simulink model "PrkgClimaEgyMgr"  (R2022b)
settings: Solver=FixedStepDiscrete  StartTime=0.0  StopTime=10.0  FixedStep=0.2  RelTol=1e-3
settings Model: Creator=blue.ge  LastModifiedBy=blue.ge
settings DataIOCC: SaveFormat=StructureWithTime
settings OptimizationCC: DefaultParameterBehavior=Inlined  EnhancedBackFolding=on  PassReuseOutputArgsAs=Individual arguments  ZeroExternalMemoryAtStartup=off  ZeroInternalMemoryAtStartup=off  OptimizeBlockOrder=speed  DifferentSizesBufferReuse=on  OptimizationCustomize=off  EfficientTunableParamExpr=on
settings DebuggingCC: ParameterTunabilityLossMsg=error
settings ModelReferenceCC: ModelReferenceNumInstancesAllowed=Single
settings SFSimCC: MATLABDynamicMemAlloc=off
settings RTWCC: Description=AUTOSAR  SystemTargetFile=autosar.tlc  GenCodeOnly=on  TemplateMakefile=ert_default_tmf
settings CodeAppCC: ForceParamTrailComments=on  IgnoreCustomStorageClasses=off  OperatorAnnotations=on  SimulinkDataObjDesc=on  SFDataObjDesc=on  InsertBlockDesc=on
settings STFCustomTargetCC: TargetLangStandard=C99 (ISO)  MultiwordLength=256  ParMdlRefBuildCompliant=on  CompOptLevelCompliant=on  IncludeMdlTerminateFcn=off  CombineOutputUpdateFcns=on  SuppressErrorStatus=on  ERTCustomFileBanners=on  SupportNonFinite=off  SupportComplex=off  SupportContinuousTime=off  SupportNonInlinedSFcns=off  RemoveResetFunc=on  MATLABClassNameForMDSCustomization=Simulink.SoftwareTarget.ERTCustomization  AutosarCompliant=on  UseToolchainInfoCompliant=on  LookupTableObjectStructAxisOrder=2,1,3,4,...  LUTObjectStructOrderEvenSpacing=Size,Table,Breakpoints  SystemTargetFile=autosar.tlc
settings ERTTargetCC: TargetLangStandard=C99 (ISO)  ConcurrentExecutionCompliant=off  IncludeMdlTerminateFcn=off  SuppressErrorStatus=on  SupportNonFinite=off  SupportComplex=off  ModelStepFunctionPrototypeControlCompliant=off  CPPClassGenCompliant=off  AutosarCompliant=on  LookupTableObjectStructAxisOrder=2,1,3,4,...  LUTObjectStructOrderEvenSpacing=Size,Table,Breakpoints  InlinedParameterPlacement=NonHierarchical  ERTCustomFileTemplate=example_file_process.tlc

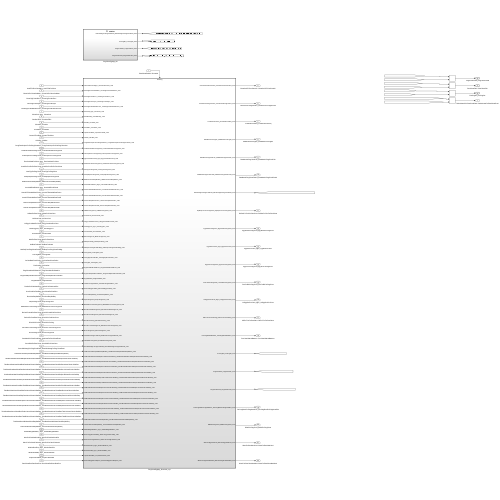
[thumbnail: Simulink's stored preview of the top-level diagram]
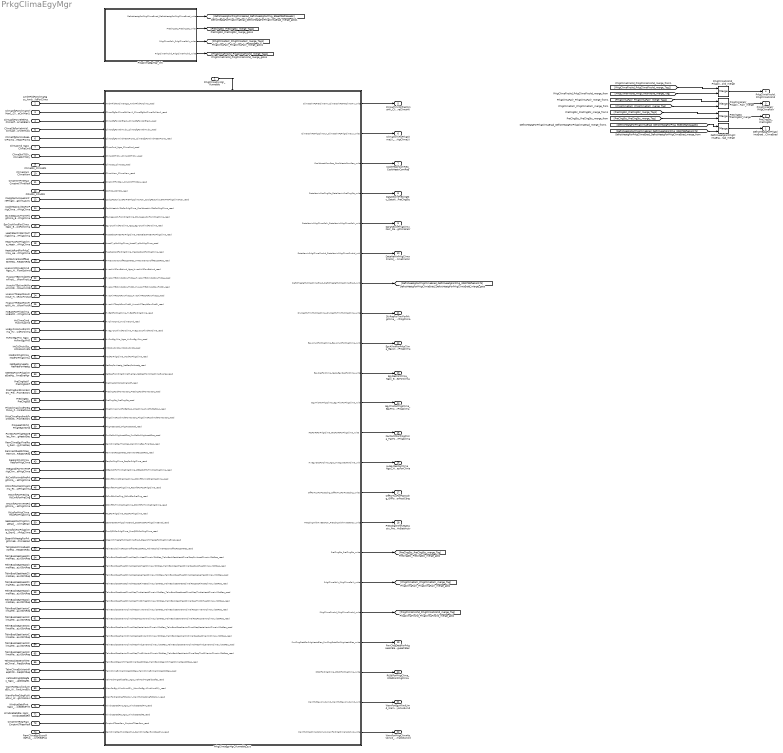
== system PrkgClimaEgyMgr ==
  PrkgClimaEgyMgr_Runnable : Inport  SampleTime=0.2  OutputFunctionCall=on
  AmbTFildForClimaNgcc_AmbTFildForClima : Inport  Port=2  OutDataTypeStr=T30  PortDimensions=1
  ClimaCfgForClimaCtrlMan1_ClimaCfgForClimaCtrlMan1 : Inport  Port=3  OutDataTypeStr=Boolean  PortDimensions=1
  ClimaCfgForVehElec1_ClimaCfgForVehElec1 : Inport  Port=4  OutDataTypeStr=Boolean  PortDimensions=1
  ClimaCfgForVehHyb1_ClimaCfgForVehHyb1 : Inport  Port=5  OutDataTypeStr=Boolean  PortDimensions=1
  ClimaCfgForWindHeatrPrsnt1_ClimaCfgForWindHeatrPrsnt1 : Inport  Port=6  OutDataTypeStr=Boolean  PortDimensions=1
  ClimaCmd_Ngcc_ClimaCmd : Inport  Port=7  OutDataTypeStr=Enum: OkNotOk  PortDimensions=1
  ClimaStrtTiDly_ClimaStrtTiDly : Inport  Port=8  OutDataTypeStr=Ti39  PortDimensions=1
  ClimaSts_ClimaSts : Inport  Port=9  OutDataTypeStr=Enum: ClimaSts  PortDimensions=1
  ClimaWarn_ClimaWarn : Inport  Port=10  OutDataTypeStr=Enum: ClimaWarn  PortDimensions=1
  CmptmtTFrntRaw_CmptmtTFrntRaw : Inport  Port=11  OutDataTypeStr=Bus: CmptmtTFrnt  PortDimensions=1  BusOutputAsStruct=on
  COHSts_COHSts : Inport  Port=12  OutDataTypeStr=Enum: CabinOHSts  PortDimensions=1
  CoolgFastUniqueForPrePrkgClimaActv_CoolgFastUniqueForPrePrkgClimaActv : Inport  Port=13  OutDataTypeStr=Boolean  PortDimensions=1
  CooltHeatrAvlStsForPrkgClima_CooltHeatrAvlStsForPrkgClima : Inport  Port=14  OutDataTypeStr=Bus: CooltHeatrAvlSts1  PortDimensions=1  BusOutputAsStruct=on
  EcuKeepActvFromPrkgClima_EcuKeepActvFromPrkgClima : Inport  Port=15  OutDataTypeStr=Boolean  PortDimensions=1
  EgyCnsAllwdForClima_Ngcc_EgyCnsAllwdForClima : Inport  Port=16  OutDataTypeStr=Egy2  PortDimensions=1
  HeatrElecFrntErrForPrkgClima_HeatrElecFrntErrForPrkgClima : Inport  Port=17  OutDataTypeStr=Boolean  PortDimensions=1
  HeatrTypForPrkgClima_HeatrTypForPrkgClima : Inport  Port=18  OutDataTypeStr=Enum: HeatrTyp  PortDimensions=1
  HeatUpRqrdForPrkgClima_HeatUpRqrdForPrkgClima : Inport  Port=19  OutDataTypeStr=Boolean  PortDimensions=1
  HMIExtVentonoffReqStrtReq_HMIExtVentonoffReqStrtReq : Inport  Port=20  OutDataTypeStr=Enum: OnOffNoReq  PortDimensions=1
  HvacAirMFlowEstimd_Ngcc_HvacAirMFlowEstimd : Inport  Port=21  OutMin=0  OutMax=1000  OutDataTypeStr=MFlow1  PortDimensions=1
  HvacAirTEstimdAtRowFirstLe_HvacAirTEstimdAtRowFirstLe : Inport  Port=22  OutDataTypeStr=T30  PortDimensions=1
  HvacAirTEstimdAtRowFirstRi_HvacAirTEstimdAtRowFirstRi : Inport  Port=23  OutDataTypeStr=T30  PortDimensions=1
  HvacAirTReqAtRowFirstLe_HvacAirTReqAtRowFirstLe : Inport  Port=24  OutDataTypeStr=T30  PortDimensions=1
  HvacAirTReqAtRowFirstRi_HvacAirTReqAtRowFirstRi : Inport  Port=25  OutDataTypeStr=T30  PortDimensions=1
  HvBattForPrkgClima_HvBattForPrkgClima : Inport  Port=26  OutDataTypeStr=Boolean  PortDimensions=1
  HvClimaCmd_HvClimaCmd : Inport  Port=27  OutDataTypeStr=Enum: HVBattChargnCmd  PortDimensions=1
  HvEgyCnsAllwdForClima_HvEgyCnsAllwdForClima : Inport  Port=28  OutDataTypeStr=HvEgyDesForClima  PortDimensions=1
  HvPwrEgyPrio_Ngcc_HvPwrEgyPrio : Inport  Port=29  OutDataTypeStr=Enum: HvPwrEgyPrio  PortDimensions=1
  IntCo2ActvSts_IntCo2ActvSts : Inport  Port=30  OutDataTypeStr=Enum: IntCo2Sts  PortDimensions=1
  ModForPrkgClima_ModForPrkgClima : Inport  Port=31  OutDataTypeStr=Enum: PrkgClimaMod  PortDimensions=1
  NetReqForHeatg_NetReqForHeatg : Inport  Port=32  OutDataTypeStr=Boolean  PortDimensions=1
  NetReqFromPrkgClimaEveMgr_NetReqFromPrkgClimaEveMgr : Inport  Port=33  OutDataTypeStr=Boolean  PortDimensions=1
  PreClngNotif_PreClngNotif : Inport  Port=34  OutDataTypeStr=Enum: PreClngNotif  PortDimensions=1
  PreClngRqrdFromExtSrc_PreClngRqrdFromExtSrc : Inport  Port=35  OutDataTypeStr=Enum: PreCdngReqFromExtSrc  PortDimensions=1
  PreClngSts_PreClngSts : Inport  Port=36  OutDataTypeStr=Enum: PreClngSts  PortDimensions=1
  PrkgClimaAllwdForBattULo_PrkgClimaAllwdForBattULo : Inport  Port=37  OutDataTypeStr=Boolean  PortDimensions=1
  PrkgClimaRqrdImdtFromExtSrc_PrkgClimaRqrdImdtFromExtSrc : Inport  Port=38  OutDataTypeStr=Enum: PreCdngReqFromExtSrc  PortDimensions=1
  PrkgHeatrStrtd_PrkgHeatrStrtd : Inport  Port=39  OutDataTypeStr=Boolean  PortDimensions=1
  PwrStsForPrkgHeatrElec_PwrStsForPrkgHeatrElec : Inport  Port=40  OutDataTypeStr=Boolean  PortDimensions=1
  RemClimaEgyFinalReq_RemClimaEgyFinalReq : Inport  Port=41  OutDataTypeStr=UInt32  PortDimensions=1
  RemVentReqStrtReq_RemVentReqStrtReq : Inport  Port=42  OutDataTypeStr=Boolean  PortDimensions=1
  ReqForPrkgClima_ReqForPrkgClima : Inport  Port=43  OutDataTypeStr=Enum: PrkgClimaReqSts  PortDimensions=1
  RtBasSdlForTmrPrePrkgClima_RtBasSdlForTmrPrePrkgClima : Inport  Port=44  OutDataTypeStr=Ti33  PortDimensions=1
  RtCmftForImdtPrePrkgClima_RtCmftForImdtPrePrkgClima : Inport  Port=45  OutDataTypeStr=Ti33  PortDimensions=1
  RtCmftForPostPrkgClima_RtCmftForPostPrkgClima : Inport  Port=46  OutDataTypeStr=Ti33  PortDimensions=1
  RtCmftForPreClng_RtCmftForPreClng : Inport  Port=47  OutDataTypeStr=Ti33  PortDimensions=1
  RtCmftForTmrPrePrkgClima_RtCmftForTmrPrePrkgClima : Inport  Port=48  OutDataTypeStr=Ti33  PortDimensions=1
  RtLeForPrkgClima_RtLeForPrkgClima : Inport  Port=49  OutDataTypeStr=Ti33  PortDimensions=1
  SeatHeatForPrkgClimaEnad_SeatHeatForPrkgClimaEnad : Inport  Port=50  OutDataTypeStr=Enum: SeatHeatDurgClimaEnad  PortDimensions=1
  SnsrQfOkForPrkgClima_SnsrQfOkForPrkgClima : Inport  Port=51  OutDataTypeStr=Boolean  PortDimensions=1
  SteerWhlHeatgForPrkgClimaEnad_SteerWhlHeatgForPrkgClimaEnad : Inport  Port=52  OutDataTypeStr=Boolean  PortDimensions=1
  TelmBookClimaReqOnOffNoReqStrtReq_TelmBookClimaReqOnOffNoReqStrtReq : Inport  Port=53  OutDataTypeStr=Enum: NoReqOnOff  PortDimensions=1
  TelmBookSeatHeatClimalReqDrvHeatClimaLvlStrtReq_TelmBookSeatHeatClimalReqDrvHeatClimaLvlStrtReq : Inport  Port=54  OutDataTypeStr=Enum: TelmSeatClimaLvl  PortDimensions=1
  TelmBookSeatHeatClimalReqPassHeatClimaLvlStrtReq_TelmBookSeatHeatClimalReqPassHeatClimaLvlStrtReq : Inport  Port=55  OutDataTypeStr=Enum: TelmSeatClimaLvl  PortDimensions=1
  TelmBookSeatHeatClimalReqSecLeHeatClimaLvlStrtReq_TelmBookSeatHeatClimalReqSecLeHeatClimaLvlStrtReq : Inport  Port=56  OutDataTypeStr=Enum: TelmSeatClimaLvl  PortDimensions=1
  TelmBookSeatHeatClimalReqSecRiHeatClimaLvlStrtReq_TelmBookSeatHeatClimalReqSecRiHeatClimaLvlStrtReq : Inport  Port=57  OutDataTypeStr=Enum: TelmSeatClimaLvl  PortDimensions=1
  TelmBookSeatHeatClimalReqThrdLeHeatClimaLvlStrtReq_TelmBookSeatHeatClimalReqThrdLeHeatClimaLvlStrtReq : Inport  Port=58  OutDataTypeStr=Enum: TelmSeatClimaLvl  PortDimensions=1
  TelmBookSeatHeatClimalReqThrdRiHeatClimaLvlStrtReq_TelmBookSeatHeatClimalReqThrdRiHeatClimaLvlStrtReq : Inport  Port=59  OutDataTypeStr=Enum: TelmSeatClimaLvl  PortDimensions=1
  TelmBookSeatVentnClimalReqDrvVentnClimaLvlStrtReq_TelmBookSeatVentnClimalReqDrvVentnClimaLvlStrtReq : Inport  Port=60  OutDataTypeStr=Enum: TelmSeatClimaLvl  PortDimensions=1
  TelmBookSeatVentnClimalReqPassVentnClimaLvlStrtReq_TelmBookSeatVentnClimalReqPassVentnClimaLvlStrtReq : Inport  Port=61  OutDataTypeStr=Enum: TelmSeatClimaLvl  PortDimensions=1
  TelmBookSeatVentnClimalReqSecLeVentnClimaLvlStrtReq_TelmBookSeatVentnClimalReqSecLeVentnClimaLvlStrtReq : Inport  Port=62  OutDataTypeStr=Enum: TelmSeatClimaLvl  PortDimensions=1
  TelmBookSeatVentnClimalReqSecRiVentnClimaLvlStrtReq_TelmBookSeatVentnClimalReqSecRiVentnClimaLvlStrtReq : Inport  Port=63  OutDataTypeStr=Enum: TelmSeatClimaLvl  PortDimensions=1
  TelmBookSeatVentnClimalReqThrdLeVentnClimaLvlStrtReq_TelmBookSeatVentnClimalReqThrdLeVentnClimaLvlStrtReq : Inport  Port=64  OutDataTypeStr=Enum: TelmSeatClimaLvl  PortDimensions=1
  TelmBookSeatVentnClimalReqThrdRiVentnClimaLvlStrtReq_TelmBookSeatVentnClimalReqThrdRiVentnClimaLvlStrtReq : Inport  Port=65  OutDataTypeStr=Enum: TelmSeatClimaLvl  PortDimensions=1
  TelmBookSteerWhlHeatClimalReqStrtReq_TelmBookSteerWhlHeatClimalReqStrtReq : Inport  Port=66  OutDataTypeStr=Enum: OnOff1  PortDimensions=1
  TelmClimaExtVentnReqStrtReq_TelmClimaExtVentnReqStrtReq : Inport  Port=67  OutDataTypeStr=Enum: OnOffNoReq  PortDimensions=1
  VehModMngtGlbSafe1_Ngcc_VehModMngtGlbSafe1 : Inport  Port=68  OutDataTypeStr=Bus: VehModMngtGlbSafe1  PortDimensions=1  BusOutputAsStruct=on
  WarnForEgyAllwdLimdDly_WarnForEgyAllwdLimdDly : Inport  Port=69  OutDataTypeStr=Boolean  PortDimensions=1
  WarnForPreCdngFctNotAvl_WarnForPreCdngFctNotAvl : Inport  Port=70  OutDataTypeStr=Boolean  PortDimensions=1
  WindIceDetdFrnt_Ngcc_WindIceDetdFrnt : Inport  Port=71  OutDataTypeStr=Enum: OnOff1  PortDimensions=1
  WindIceDetdRe_Ngcc_WindIceDetdRe : Inport  Port=72  OutDataTypeStr=Enum: OnOff1  PortDimensions=1
  CmptmtTRearRaw_CmptmtTRearRaw : Inport  Port=73  OutDataTypeStr=Bus: CmptmtTFrnt  PortDimensions=1  BusOutputAsStruct=on
  RemClimaEgyExsrcReqPlus_RemClimaEgyExsrcReqPlus : Inport  Port=74  OutDataTypeStr=UInt32  PortDimensions=1
  DefrstHeatgForPrkgClimaEnad_DefrstHeatgForPrkgClimaEnad_merge : Merge
  PreClngStc_PreClngStc_merge : Merge
  PrkgClimaEgyMgr_Init : Subsystem
  PrkgClimaEgyMgr_Runnable_sys : Subsystem
  PrkgClimaFailr_PrkgClimaFailr_merge : Merge
  PrkgClimaFinshd_PrkgClimaFinshd_merge : Merge
  DefrstHeatgForPrkgClimaEnad_DefrstHeatgForPrkgClimaEnad : Outport  OutDataTypeStr=Boolean  PortDimensions=1
  PreClngStc_PreClngStc : Outport  Port=2  OutDataTypeStr=Bus: PreClngStcTyp  PortDimensions=1  BusOutputAsStruct=on
  PrkgClimaFailr_PrkgClimaFailr : Outport  Port=3  OutDataTypeStr=Bus: PrkgClimaFailrTyp  PortDimensions=1  BusOutputAsStruct=on
  PrkgClimaFinshd_PrkgClimaFinshd : Outport  Port=4  OutDataTypeStr=Bus: PrkgClimaFinshdTyp  PortDimensions=1  BusOutputAsStruct=on
  ClimaCtrlVFCPreClimaHV_ClimaCtrlVFCPreClimaHV : Outport  Port=5  OutDataTypeStr=Enum: OnOff1  PortDimensions=1
  ClimaCtrlVFCPrkgClimaLV_ClimaCtrlVFCPrkgClimaLV : Outport  Port=6  OutDataTypeStr=Enum: OnOff1  PortDimensions=1
  CooltHeatrComReq_CooltHeatrComReq : Outport  Port=7  OutDataTypeStr=Boolean  PortDimensions=1
  DataNewInPreClngStc_DataNewInPreClngStc : Outport  Port=8  OutDataTypeStr=Boolean  PortDimensions=1
  DataNewInPrkgClimaFailr_DataNewInPrkgClimaFailr : Outport  Port=9  OutDataTypeStr=Boolean  PortDimensions=1
  DataNewInPrkgClimaFinshd_DataNewInPrkgClimaFinshd : Outport  Port=10  OutDataTypeStr=Boolean  PortDimensions=1
  DlyReqForTmrForPrkgClima_DlyReqForTmrForPrkgClima : Outport  Port=11  OutDataTypeStr=Boolean  PortDimensions=1
  EgyAllwdForPrkgClima_EgyAllwdForPrkgClima : Outport  Port=12  OutDataTypeStr=Boolean  PortDimensions=1
  EgyDesForClima_Ngcc_EgyDesForClima : Outport  Port=13  OutDataTypeStr=Egy2  PortDimensions=1
  EgyPrioForPrkgClima_EgyPrioForPrkgClima : Outport  Port=14  OutDataTypeStr=Enum: EgyPrio  PortDimensions=1
  FacForRtForPrkgClima_FacForRtForPrkgClima : Outport  Port=15  OutDataTypeStr=Fac30  PortDimensions=1
  HvEgyDesForClima_Ngcc_HvEgyDesForClima : Outport  Port=16  OutDataTypeStr=HvEgyDesForClima  PortDimensions=1
  OffForTLoForPostCdng_OffForTLoForPostCdng : Outport  Port=17  OutDataTypeStr=Boolean  PortDimensions=1
  PreCdngWithHvBattActv_PreCdngWithHvBattActv : Outport  Port=18  OutDataTypeStr=Boolean  PortDimensions=1
  PwrChrgDetdForPrkgHeatrElec_PwrChrgDetdForPrkgHeatrElec : Outport  Port=19  OutDataTypeStr=Boolean  PortDimensions=1
  RtSdlForPrkgClima_RtSdlForPrkgClima : Outport  Port=20  OutDataTypeStr=Ti33  PortDimensions=1
  WarnForEgyAllwdLimd_WarnForEgyAllwdLimd : Outport  Port=21  OutDataTypeStr=Boolean  PortDimensions=1
  WarnForPrkgClimaNotAllwd_WarnForPrkgClimaNotAllwd : Outport  Port=22  OutDataTypeStr=Enum: ClimaWarn  PortDimensions=1
  wires:
  AmbTFildForClimaNgcc_AmbTFildForClima/1 -> PrkgClimaEgyMgr_Runnable_sys/1(AmbTFildForClimaNgcc_AmbTFildForClima_read)
  ClimaCfgForClimaCtrlMan1_ClimaCfgForClimaCtrlMan1/1 -> PrkgClimaEgyMgr_Runnable_sys/2(ClimaCfgForClimaCtrlMan1_ClimaCfgForClimaCtrlMan1_read)
  ClimaCfgForVehElec1_ClimaCfgForVehElec1/1 -> PrkgClimaEgyMgr_Runnable_sys/3(ClimaCfgForVehElec1_ClimaCfgForVehElec1_read)
  ClimaCfgForVehHyb1_ClimaCfgForVehHyb1/1 -> PrkgClimaEgyMgr_Runnable_sys/4(ClimaCfgForVehHyb1_ClimaCfgForVehHyb1_read)
  ClimaCfgForWindHeatrPrsnt1_ClimaCfgForWindHeatrPrsnt1/1 -> PrkgClimaEgyMgr_Runnable_sys/5(ClimaCfgForWindHeatrPrsnt1_ClimaCfgForWindHeatrPrsnt1_read)
  ClimaCmd_Ngcc_ClimaCmd/1 -> PrkgClimaEgyMgr_Runnable_sys/6(ClimaCmd_Ngcc_ClimaCmd_read)
  ClimaStrtTiDly_ClimaStrtTiDly/1 -> PrkgClimaEgyMgr_Runnable_sys/7(ClimaStrtTiDly_ClimaStrtTiDly_read)
  ClimaSts_ClimaSts/1 -> PrkgClimaEgyMgr_Runnable_sys/8(ClimaSts_ClimaSts_read)
  ClimaWarn_ClimaWarn/1 -> PrkgClimaEgyMgr_Runnable_sys/9(ClimaWarn_ClimaWarn_read)
  CmptmtTFrntRaw_CmptmtTFrntRaw/1 -> PrkgClimaEgyMgr_Runnable_sys/10(CmptmtTFrntRaw_CmptmtTFrntRaw_read)
  COHSts_COHSts/1 -> PrkgClimaEgyMgr_Runnable_sys/11(COHSts_COHSts_read)
  CoolgFastUniqueForPrePrkgClimaActv_CoolgFastUniqueForPrePrkgClimaActv/1 -> PrkgClimaEgyMgr_Runnable_sys/12(CoolgFastUniqueForPrePrkgClimaActv_CoolgFastUniqueForPrePrkgClimaActv_read)
  CooltHeatrAvlStsForPrkgClima_CooltHeatrAvlStsForPrkgClima/1 -> PrkgClimaEgyMgr_Runnable_sys/13(CooltHeatrAvlStsForPrkgClima_CooltHeatrAvlStsForPrkgClima_read)
  EcuKeepActvFromPrkgClima_EcuKeepActvFromPrkgClima/1 -> PrkgClimaEgyMgr_Runnable_sys/14(EcuKeepActvFromPrkgClima_EcuKeepActvFromPrkgClima_read)
  EgyCnsAllwdForClima_Ngcc_EgyCnsAllwdForClima/1 -> PrkgClimaEgyMgr_Runnable_sys/15(EgyCnsAllwdForClima_Ngcc_EgyCnsAllwdForClima_read)
  HeatrElecFrntErrForPrkgClima_HeatrElecFrntErrForPrkgClima/1 -> PrkgClimaEgyMgr_Runnable_sys/16(HeatrElecFrntErrForPrkgClima_HeatrElecFrntErrForPrkgClima_read)
  HeatrTypForPrkgClima_HeatrTypForPrkgClima/1 -> PrkgClimaEgyMgr_Runnable_sys/17(HeatrTypForPrkgClima_HeatrTypForPrkgClima_read)
  HeatUpRqrdForPrkgClima_HeatUpRqrdForPrkgClima/1 -> PrkgClimaEgyMgr_Runnable_sys/18(HeatUpRqrdForPrkgClima_HeatUpRqrdForPrkgClima_read)
  HMIExtVentonoffReqStrtReq_HMIExtVentonoffReqStrtReq/1 -> PrkgClimaEgyMgr_Runnable_sys/19(HMIExtVentonoffReqStrtReq_HMIExtVentonoffReqStrtReq_read)
  HvacAirMFlowEstimd_Ngcc_HvacAirMFlowEstimd/1 -> PrkgClimaEgyMgr_Runnable_sys/20(HvacAirMFlowEstimd_Ngcc_HvacAirMFlowEstimd_read)
  HvacAirTEstimdAtRowFirstLe_HvacAirTEstimdAtRowFirstLe/1 -> PrkgClimaEgyMgr_Runnable_sys/21(HvacAirTEstimdAtRowFirstLe_HvacAirTEstimdAtRowFirstLe_read)
  HvacAirTEstimdAtRowFirstRi_HvacAirTEstimdAtRowFirstRi/1 -> PrkgClimaEgyMgr_Runnable_sys/22(HvacAirTEstimdAtRowFirstRi_HvacAirTEstimdAtRowFirstRi_read)
  HvacAirTReqAtRowFirstLe_HvacAirTReqAtRowFirstLe/1 -> PrkgClimaEgyMgr_Runnable_sys/23(HvacAirTReqAtRowFirstLe_HvacAirTReqAtRowFirstLe_read)
  HvacAirTReqAtRowFirstRi_HvacAirTReqAtRowFirstRi/1 -> PrkgClimaEgyMgr_Runnable_sys/24(HvacAirTReqAtRowFirstRi_HvacAirTReqAtRowFirstRi_read)
  HvBattForPrkgClima_HvBattForPrkgClima/1 -> PrkgClimaEgyMgr_Runnable_sys/25(HvBattForPrkgClima_HvBattForPrkgClima_read)
  HvClimaCmd_HvClimaCmd/1 -> PrkgClimaEgyMgr_Runnable_sys/26(HvClimaCmd_HvClimaCmd_read)
  HvEgyCnsAllwdForClima_HvEgyCnsAllwdForClima/1 -> PrkgClimaEgyMgr_Runnable_sys/27(HvEgyCnsAllwdForClima_HvEgyCnsAllwdForClima_read)
  HvPwrEgyPrio_Ngcc_HvPwrEgyPrio/1 -> PrkgClimaEgyMgr_Runnable_sys/28(HvPwrEgyPrio_Ngcc_HvPwrEgyPrio_read)
  IntCo2ActvSts_IntCo2ActvSts/1 -> PrkgClimaEgyMgr_Runnable_sys/29(IntCo2ActvSts_IntCo2ActvSts_read)
  ModForPrkgClima_ModForPrkgClima/1 -> PrkgClimaEgyMgr_Runnable_sys/30(ModForPrkgClima_ModForPrkgClima_read)
  NetReqForHeatg_NetReqForHeatg/1 -> PrkgClimaEgyMgr_Runnable_sys/31(NetReqForHeatg_NetReqForHeatg_read)
  NetReqFromPrkgClimaEveMgr_NetReqFromPrkgClimaEveMgr/1 -> PrkgClimaEgyMgr_Runnable_sys/32(NetReqFromPrkgClimaEveMgr_NetReqFromPrkgClimaEveMgr_read)
  PreClngNotif_PreClngNotif/1 -> PrkgClimaEgyMgr_Runnable_sys/33(PreClngNotif_PreClngNotif_read)
  PreClngRqrdFromExtSrc_PreClngRqrdFromExtSrc/1 -> PrkgClimaEgyMgr_Runnable_sys/34(PreClngRqrdFromExtSrc_PreClngRqrdFromExtSrc_read)
  PreClngSts_PreClngSts/1 -> PrkgClimaEgyMgr_Runnable_sys/35(PreClngSts_PreClngSts_read)
  PrkgClimaAllwdForBattULo_PrkgClimaAllwdForBattULo/1 -> PrkgClimaEgyMgr_Runnable_sys/36(PrkgClimaAllwdForBattULo_PrkgClimaAllwdForBattULo_read)
  PrkgClimaRqrdImdtFromExtSrc_PrkgClimaRqrdImdtFromExtSrc/1 -> PrkgClimaEgyMgr_Runnable_sys/37(PrkgClimaRqrdImdtFromExtSrc_PrkgClimaRqrdImdtFromExtSrc_read)
  PrkgHeatrStrtd_PrkgHeatrStrtd/1 -> PrkgClimaEgyMgr_Runnable_sys/38(PrkgHeatrStrtd_PrkgHeatrStrtd_read)
  PwrStsForPrkgHeatrElec_PwrStsForPrkgHeatrElec/1 -> PrkgClimaEgyMgr_Runnable_sys/39(PwrStsForPrkgHeatrElec_PwrStsForPrkgHeatrElec_read)
  RemClimaEgyFinalReq_RemClimaEgyFinalReq/1 -> PrkgClimaEgyMgr_Runnable_sys/40(RemClimaEgyFinalReq_RemClimaEgyFinalReq_read)
  RemVentReqStrtReq_RemVentReqStrtReq/1 -> PrkgClimaEgyMgr_Runnable_sys/41(RemVentReqStrtReq_RemVentReqStrtReq_read)
  ReqForPrkgClima_ReqForPrkgClima/1 -> PrkgClimaEgyMgr_Runnable_sys/42(ReqForPrkgClima_ReqForPrkgClima_read)
  RtBasSdlForTmrPrePrkgClima_RtBasSdlForTmrPrePrkgClima/1 -> PrkgClimaEgyMgr_Runnable_sys/43(RtBasSdlForTmrPrePrkgClima_RtBasSdlForTmrPrePrkgClima_read)
  RtCmftForImdtPrePrkgClima_RtCmftForImdtPrePrkgClima/1 -> PrkgClimaEgyMgr_Runnable_sys/44(RtCmftForImdtPrePrkgClima_RtCmftForImdtPrePrkgClima_read)
  RtCmftForPostPrkgClima_RtCmftForPostPrkgClima/1 -> PrkgClimaEgyMgr_Runnable_sys/45(RtCmftForPostPrkgClima_RtCmftForPostPrkgClima_read)
  RtCmftForPreClng_RtCmftForPreClng/1 -> PrkgClimaEgyMgr_Runnable_sys/46(RtCmftForPreClng_RtCmftForPreClng_read)
  RtCmftForTmrPrePrkgClima_RtCmftForTmrPrePrkgClima/1 -> PrkgClimaEgyMgr_Runnable_sys/47(RtCmftForTmrPrePrkgClima_RtCmftForTmrPrePrkgClima_read)
  RtLeForPrkgClima_RtLeForPrkgClima/1 -> PrkgClimaEgyMgr_Runnable_sys/48(RtLeForPrkgClima_RtLeForPrkgClima_read)
  SeatHeatForPrkgClimaEnad_SeatHeatForPrkgClimaEnad/1 -> PrkgClimaEgyMgr_Runnable_sys/49(SeatHeatForPrkgClimaEnad_SeatHeatForPrkgClimaEnad_read)
  SnsrQfOkForPrkgClima_SnsrQfOkForPrkgClima/1 -> PrkgClimaEgyMgr_Runnable_sys/50(SnsrQfOkForPrkgClima_SnsrQfOkForPrkgClima_read)
  SteerWhlHeatgForPrkgClimaEnad_SteerWhlHeatgForPrkgClimaEnad/1 -> PrkgClimaEgyMgr_Runnable_sys/51(SteerWhlHeatgForPrkgClimaEnad_SteerWhlHeatgForPrkgClimaEnad_read)
  TelmBookClimaReqOnOffNoReqStrtReq_TelmBookClimaReqOnOffNoReqStrtReq/1 -> PrkgClimaEgyMgr_Runnable_sys/52(TelmBookClimaReqOnOffNoReqStrtReq_TelmBookClimaReqOnOffNoReqStrtReq_read)
  TelmBookSeatHeatClimalReqDrvHeatClimaLvlStrtReq_TelmBookSeatHeatClimalReqDrvHeatClimaLvlStrtReq/1 -> PrkgClimaEgyMgr_Runnable_sys/53(TelmBookSeatHeatClimalReqDrvHeatClimaLvlStrtReq_TelmBookSeatHeatClimalReqDrvHeatClimaLvlStrtReq_read)
  TelmBookSeatHeatClimalReqPassHeatClimaLvlStrtReq_TelmBookSeatHeatClimalReqPassHeatClimaLvlStrtReq/1 -> PrkgClimaEgyMgr_Runnable_sys/54(TelmBookSeatHeatClimalReqPassHeatClimaLvlStrtReq_TelmBookSeatHeatClimalReqPassHeatClimaLvlStrtReq_read)
  TelmBookSeatHeatClimalReqSecLeHeatClimaLvlStrtReq_TelmBookSeatHeatClimalReqSecLeHeatClimaLvlStrtReq/1 -> PrkgClimaEgyMgr_Runnable_sys/55(TelmBookSeatHeatClimalReqSecLeHeatClimaLvlStrtReq_TelmBookSeatHeatClimalReqSecLeHeatClimaLvlStrtReq_read)
  TelmBookSeatHeatClimalReqSecRiHeatClimaLvlStrtReq_TelmBookSeatHeatClimalReqSecRiHeatClimaLvlStrtReq/1 -> PrkgClimaEgyMgr_Runnable_sys/56(TelmBookSeatHeatClimalReqSecRiHeatClimaLvlStrtReq_TelmBookSeatHeatClimalReqSecRiHeatClimaLvlStrtReq_read)
  TelmBookSeatHeatClimalReqThrdLeHeatClimaLvlStrtReq_TelmBookSeatHeatClimalReqThrdLeHeatClimaLvlStrtReq/1 -> PrkgClimaEgyMgr_Runnable_sys/57(TelmBookSeatHeatClimalReqThrdLeHeatClimaLvlStrtReq_TelmBookSeatHeatClimalReqThrdLeHeatClimaLvlStrtReq_read)
  TelmBookSeatHeatClimalReqThrdRiHeatClimaLvlStrtReq_TelmBookSeatHeatClimalReqThrdRiHeatClimaLvlStrtReq/1 -> PrkgClimaEgyMgr_Runnable_sys/58(TelmBookSeatHeatClimalReqThrdRiHeatClimaLvlStrtReq_TelmBookSeatHeatClimalReqThrdRiHeatClimaLvlStrtReq_read)
  TelmBookSeatVentnClimalReqDrvVentnClimaLvlStrtReq_TelmBookSeatVentnClimalReqDrvVentnClimaLvlStrtReq/1 -> PrkgClimaEgyMgr_Runnable_sys/59(TelmBookSeatVentnClimalReqDrvVentnClimaLvlStrtReq_TelmBookSeatVentnClimalReqDrvVentnClimaLvlStrtReq_read)
  TelmBookSeatVentnClimalReqPassVentnClimaLvlStrtReq_TelmBookSeatVentnClimalReqPassVentnClimaLvlStrtReq/1 -> PrkgClimaEgyMgr_Runnable_sys/60(TelmBookSeatVentnClimalReqPassVentnClimaLvlStrtReq_TelmBookSeatVentnClimalReqPassVentnClimaLvlStrtReq_read)
  TelmBookSeatVentnClimalReqSecLeVentnClimaLvlStrtReq_TelmBookSeatVentnClimalReqSecLeVentnClimaLvlStrtReq/1 -> PrkgClimaEgyMgr_Runnable_sys/61(TelmBookSeatVentnClimalReqSecLeVentnClimaLvlStrtReq_TelmBookSeatVentnClimalReqSecLeVentnClimaLvlStrtReq_read)
  TelmBookSeatVentnClimalReqSecRiVentnClimaLvlStrtReq_TelmBookSeatVentnClimalReqSecRiVentnClimaLvlStrtReq/1 -> PrkgClimaEgyMgr_Runnable_sys/62(TelmBookSeatVentnClimalReqSecRiVentnClimaLvlStrtReq_TelmBookSeatVentnClimalReqSecRiVentnClimaLvlStrtReq_read)
  TelmBookSeatVentnClimalReqThrdLeVentnClimaLvlStrtReq_TelmBookSeatVentnClimalReqThrdLeVentnClimaLvlStrtReq/1 -> PrkgClimaEgyMgr_Runnable_sys/63(TelmBookSeatVentnClimalReqThrdLeVentnClimaLvlStrtReq_TelmBookSeatVentnClimalReqThrdLeVentnClimaLvlStrtReq_read)
  TelmBookSeatVentnClimalReqThrdRiVentnClimaLvlStrtReq_TelmBookSeatVentnClimalReqThrdRiVentnClimaLvlStrtReq/1 -> PrkgClimaEgyMgr_Runnable_sys/64(TelmBookSeatVentnClimalReqThrdRiVentnClimaLvlStrtReq_TelmBookSeatVentnClimalReqThrdRiVentnClimaLvlStrtReq_read)
  TelmBookSteerWhlHeatClimalReqStrtReq_TelmBookSteerWhlHeatClimalReqStrtReq/1 -> PrkgClimaEgyMgr_Runnable_sys/65(TelmBookSteerWhlHeatClimalReqStrtReq_TelmBookSteerWhlHeatClimalReqStrtReq_read)
  TelmClimaExtVentnReqStrtReq_TelmClimaExtVentnReqStrtReq/1 -> PrkgClimaEgyMgr_Runnable_sys/66(TelmClimaExtVentnReqStrtReq_TelmClimaExtVentnReqStrtReq_read)